simulink model "TosAeb"  (R2021a)
settings: Solver=FixedStepDiscrete  StartTime=0.0  StopTime=inf  FixedStep=0.01  RelTol=1e-3
undefined symbols (not defined in the file or in its folder): ACCModSts, AccSts1, AEB_ObjectMovingOrientation, AEB_ObjectMovingState, CN_BTCActive, CN_CurrentGearR, CN_CurrentGearR_DHT, CN_PTCActive, CN_VDCActive, CN_VSC_EspOff, CN_VSC_FCW_AEB_Error, CN_VSC_FCW_AEB_NoDispay, CN_VSC_FCW_AEB_PerfmDgdtn, CN_VSC_FCW_AEB_TempUnAvlbl, CN_VSC_VehStandstill, CNaccelAvoidMax, CNaccelAvoidMin, CNbrakePedalPress, CNcomfortStrLeftAccelMax, CNcomfortStrLeftAccelMin, CNcomfortStrRightAccelMax, CNcomfortStrRightAccelMin, CNDrvDoorOpen, CNDrvModeEco, CNDrvModeNorm, CNDrvModeOther, CNDrvModeSport, CNDrvSBRreminder, CNNull, CNobjInvalidID, CNPassDoorOpen, CNPassSBRreminder, CNPEBSAccelNegInf, CNPEBSAccelNull, CNPEBSTimeInf, CNPEBSTimeNull, CNSTOPMAX, CNSTOPNULL, CNsubjWidth, CNSwtOFF, CNSwtON, CNTTCMAX, CNTTCNULL, CNVehConfigAEBCycNone, CNVehConfigAEBNone, CNVehConfigAEBPedNone, CNVehConfigFCWNone, CNVehConfigJANone, CollisionSeverity, FALSE, FCWSnvty, JACycObjCalcInfoDefaultParams, JAPedObjCalcInfoDefaultParams, JAVehObjCalcInfoDefaultParams, latCycObjCalcInfoDefaultParams, latPedObjCalcInfoDefaultParams, longObjCalcInfoDefaultParams, ModSts, movingStatus, ObjectType ... (+1112)
libraries: simulink x3031, AEBSFunctionLib x50, Ta_Library x33, AEBSStateMachineLib x23, Mylibrary x12, simulink_extras x7, ObjSelect_lib x3, dspstat3 x2
(part 23 of 102)

== system TosAeb/FcwAebJa_Step_sys/ALG_AEBSystem/ALG_AEBController/AEB_FuncCondCalcApp/JaPedFunctionCondCalc/AEBJAPedAEBfullSuppAborCond/AEBJAPedAEBfullAborCondCalc ==
  inputSignals : Inport
  AEBJAPedAEBfullAborCondFinalJudge : Subsystem
  AEBJAPedAEBfullObjRelAborCondCalc : Subsystem
  AEBJAPedAEBfullVehRelAborCondCalc : Subsystem
  Bus Selector : BusSelector  OutputSignals=AEBJAPedTgtCalcInfo.JAPedTgtObjInfo.AEBJAPedObj.ObjectID
  Bus Selector1 : BusSelector  OutputSignals=vehCanInfo.SteerWheelSpd,vehCanInfo.AccrPedlPosnDiagc,vehStateCalcInfo.VSC_JAFuncSuppAborCond.VSC_JAPedVehAEBfullAborCond
  AEBJAPedAEBfullAborCondNoStandstillFulfilled : Outport
  AEBJAPedAEBfullAborCondStandstillFulfilled : Outport  Port=2
  wires:
  Bus Selector1/2 -> AEBJAPedAEBfullVehRelAborCondCalc/2(AccelPedalPositionDiag)  "<AccrPedlPosnDiagc>"
  Bus Selector1/1 -> AEBJAPedAEBfullVehRelAborCondCalc/1(SteerWheelSpd_DegS)  "<SteerWheelSpd>"
  AEBJAPedAEBfullAborCondFinalJudge/1(AEBJAPedAEBfullAborCondNoStndstillFulfilled) -> AEBJAPedAEBfullAborCondNoStandstillFulfilled/1
  AEBJAPedAEBfullVehRelAborCondCalc/1(AEBJAPedAEBfullVehRelAborCondFulfilled) -> AEBJAPedAEBfullAborCondFinalJudge/2(AEBJAPedAEBfullVehRelAborCondFulfilled)  "AEB_JaPedAEBfullVehRelAborCond"
  AEBJAPedAEBfullObjRelAborCondCalc/1(AEBJAPedAEBfullObjRelAborCondFulfilled) -> AEBJAPedAEBfullAborCondFinalJudge/1(AEBJAPedAEBfullObjRelAborCondFulfilled)  "AEB_JAPedAEBfullObjRelAborCond"
  inputSignals/1 -> Bus Selector1/1, Bus Selector/1
  Bus Selector/1 -> AEBJAPedAEBfullObjRelAborCondCalc/1(ObjectID)  "<ObjectID>"
  Bus Selector1/3 -> AEBJAPedAEBfullVehRelAborCondCalc/3(VSC_JAPedAEBfullAborCond)  "<VSC_JAPedVehAEBfullAborCond>"
  AEBJAPedAEBfullAborCondFinalJudge/2(AEBJAPedAEBfullAborCondStndstillFulfilled) -> AEBJAPedAEBfullAborCondStandstillFulfilled/1

== system TosAeb/FcwAebJa_Step_sys/ALG_AEBSystem/ALG_AEBController/AEB_FuncCondCalcApp/JaPedFunctionCondCalc/AEBJAPedAEBfullSuppAborCond/AEBJAPedAEBfullSuppCondCalc ==
  inputSignals : Inport
  AEBJAPedAEBfullSuppCondFinalJudge : Subsystem
  AEBJAPedAEBfullSuppObjCondCalcu : Subsystem
  AEBJAPedAEBfullSuppSituationCondCalc : Subsystem
  AEBJAPedAEBfullSuppVehCondCalc : Subsystem
  Bus Selector : BusSelector  OutputSignals=AEBJAPedTgtCalcInfo.JAPedTgtObjInfo.AEBJAPedObj.ObjectMovingOrientation,AEBJAPedTgtCalcInfo.JAPedTgtObjInfo.AEBJAPedObj.ObjectID,AEBJAPedTgtCalcInfo.JAPedTgtObjInfo.AEBJAPedObj.ObjectExistProb,AEBJAPedTgtCalcInfo.JAPedTgtObjInfo.AEBJAPedObj.ObjectIsObstacleProb,AEBJAPedTgtCalcInfo.JAPedTgtObjInfo.AEBJAPedObj.ObjectTypeCameraConfirmed,AEBJAPedTgtCalcInfo.JAPedTgtObjInfo.AEBJAPedObj.dy,AEBJAPedTgtCalcInfo.JAPedTgtObjInfo.AEBJAPedObjAbsInfo.vxAbsObj,AEBJAPedTgtCalcInfo.JAPedTgtObjInfo.AEBJAPedObjAbsInfo.vyRelCorr,AEBJAPedTgtCalcInfo.JAPedTgtObjInfo.AEBJAPedObjAbsInfo.ObjFusQuality
  Bus Selector1 : BusSelector  OutputSignals=subjInfo.subjVel,vehConfigInfo.VehConfigIbooster,vehCanInfo.ESP_MasterCylindBrakePress,vehCanInfo.AccrPedlPosnDiagc,vehCanInfo.SteerWheelAngle_Deg,vehCanInfo.LeftTurnSWSts,vehCanInfo.RightTurnSWSts,subjInfo.subjLatAccel,vehStateCalcInfo.VSC_JAFuncSuppAborCond.VSC_JAPedAEBfullSuppCond
  Bus Selector2 : BusSelector  OutputSignals=AEBJAPedTgtCalcInfo.JAPedCollisonAssessInfo.distBraking,AEBJAPedTgtCalcInfo.JAPedCollisonAssessInfo.distRadical2Collsion,AEBJAPedTgtCalcInfo.JAPedTgtObjInfo.AEBJAPedObjColliInfo.tPredictTime,AEBJAPedTgtCalcInfo.JAPedCollisonAssessInfo.TTB,AEBJAPedTgtCalcInfo.JAPedTgtObjInfo.AEBJAPedObjColliInfo.ObjColliFlg
  AEBJAPedAEBfullSuppCondFulfilled : Outport
  wires:
  Bus Selector1/2 -> AEBJAPedAEBfullSuppVehCondCalc/2(VehConfigIbooster)  "<VehConfigIbooster>"
  Bus Selector/9 -> AEBJAPedAEBfullSuppObjCondCalcu/9(ObjFusQuality)  "<ObjFusQuality>"
  Bus Selector/6 -> AEBJAPedAEBfullSuppObjCondCalcu/6(dy)  "<dy>"
  Bus Selector2/5 -> AEBJAPedAEBfullSuppSituationCondCalc/5(ObjColliFlg)  "<ObjColliFlg>"
  Bus Selector2/1 -> AEBJAPedAEBfullSuppSituationCondCalc/1(distBraking)  "<distBraking>"
  Bus Selector2/4 -> AEBJAPedAEBfullSuppSituationCondCalc/4(TTB)  "<TTB>"
  Bus Selector2/2 -> AEBJAPedAEBfullSuppSituationCondCalc/2(distRadical2Collsion)  "<distRadical2Collsion>"
  Bus Selector2/3 -> AEBJAPedAEBfullSuppSituationCondCalc/3(tPredictTime)  "<tPredictTime>"
  Bus Selector1/7 -> AEBJAPedAEBfullSuppVehCondCalc/7(RightTurnSWSts)  "<RightTurnSWSts>"
  Bus Selector1/8 -> AEBJAPedAEBfullSuppVehCondCalc/8(subjLatAccel)  "<subjLatAccel>"
  Bus Selector1/6 -> AEBJAPedAEBfullSuppVehCondCalc/6(LeftTurnSWSts)  "<LeftTurnSWSts>"
  Bus Selector1/5 -> AEBJAPedAEBfullSuppVehCondCalc/5(SteerWheelAngle_Deg)  "<SteerWheelAngle_Deg>"
  Bus Selector1/4 -> AEBJAPedAEBfullSuppVehCondCalc/4(AccrPedlPosnDiagc)  "<AccrPedlPosnDiagc>"
  Bus Selector1/3 -> AEBJAPedAEBfullSuppVehCondCalc/3(ESP_MasterCylindBrakePress)  "<ESP_MasterCylindBrakePress>"
  Bus Selector1/1 -> AEBJAPedAEBfullSuppVehCondCalc/1(subjVel)  "<subjVel>"
  Bus Selector/8 -> AEBJAPedAEBfullSuppObjCondCalcu/8(vyRelCorr)  "<vyRelCorr>"
  Bus Selector/7 -> AEBJAPedAEBfullSuppObjCondCalcu/7(vxAbsObj)  "<vxAbsObj>"
  Bus Selector/5 -> AEBJAPedAEBfullSuppObjCondCalcu/5(ObjectTypeCameraConfirmed)  "<ObjectTypeCameraConfirmed>"
  Bus Selector/4 -> AEBJAPedAEBfullSuppObjCondCalcu/4(ObjectIsObstacleProb)  "<ObjectIsObstacleProb>"
  Bus Selector/3 -> AEBJAPedAEBfullSuppObjCondCalcu/3(ObjectExistProb)  "<ObjectExistProb>"
  Bus Selector/2 -> AEBJAPedAEBfullSuppObjCondCalcu/2(ObjectID)  "<ObjectID>"
  Bus Selector/1 -> AEBJAPedAEBfullSuppObjCondCalcu/1(ObjectMovingOrientation)  "<ObjectMovingOrientation>"
  AEBJAPedAEBfullSuppSituationCondCalc/1(AEBJAPedAEBfullSuppSituationActiveCondFulfilled) -> AEBJAPedAEBfullSuppCondFinalJudge/3(AEBJAPedAEBfullSituationRelActiveCondFulfilled)  "AEB_JAPedAEBfullSitActiveCond"
  AEBJAPedAEBfullSuppObjCondCalcu/1(AEBJAPedAEBfullObjActiveCondFulfilled) -> AEBJAPedAEBfullSuppCondFinalJudge/1(AEBJAPedAEBfullObjRelActiveCondFulfilled)  "AEB_JaPedAEBfullObjActiveCond"
  AEBJAPedAEBfullSuppVehCondCalc/1(AEBJAPedAEBfullVehActiveCondFulfilled) -> AEBJAPedAEBfullSuppCondFinalJudge/2(AEBJAPedAEBfullVehRelActiveCondFulfilled)  "AEB_JaPedAEBfullVehActiveCond"
  AEBJAPedAEBfullSuppCondFinalJudge/1(AEBJAPedAEBfullSuppCondFulfilled) -> AEBJAPedAEBfullSuppCondFulfilled/1
  inputSignals/1 -> Bus Selector/1, Bus Selector1/1, Bus Selector2/1
  Bus Selector1/9 -> AEBJAPedAEBfullSuppVehCondCalc/9(VSC_JAPedAEBfullSuppCond)  "<VSC_JAPedAEBfullSuppCond>"

== system TosAeb/FcwAebJa_Step_sys/ALG_AEBSystem/ALG_AEBController/AEB_FuncCondCalcApp/JaPedFunctionCondCalc/AEBJAPedPrefillSuppAborCond/AEBJAPedPrefillAborCondCalc ==
  inputSignals : Inport
  AEBJAPedPrefillAborCondFinalJudge : Subsystem
  AEBJAPedPrefillObjRelAborCondCalc : Subsystem
  AEBJAPedPrefillSituationRelAborCondCalc : Subsystem
  AEBJAPedPrefillVehRelAborCondCalc : Subsystem
  Bus Selector : BusSelector  OutputSignals=AEBJAPedTgtCalcInfo.JAPedTgtObjInfo.AEBJAPedObj.ObjectID
  Bus Selector1 : BusSelector  OutputSignals=vehCanInfo.SteerWheelSpd_DegS,vehCanInfo.AccrPedlPosnDiagc,vehStateCalcInfo.VSC_JAFuncSuppAborCond.VSC_JAPedPrefillAborCond
  AEBJAPedPrefillAborCondFulfilled : Outport
  wires:
  Bus Selector1/3 -> AEBJAPedPrefillVehRelAborCondCalc/3(VSC_JAPedPrefillAborCond)  "<VSC_JAPedPrefillAborCond>"
  Bus Selector/1 -> AEBJAPedPrefillObjRelAborCondCalc/1(ObjectID)  "<ObjectID>"
  inputSignals/1 -> Bus Selector/1, Bus Selector1/1
  AEBJAPedPrefillObjRelAborCondCalc/1(AEBJAPedPrefillObjRelAborCondFulfilled) -> AEBJAPedPrefillAborCondFinalJudge/1(AEBJAPedPrefillObjRelAborCondFulfilled)  "AEB_JaPedPrefillObjRelAborCond"
  AEBJAPedPrefillVehRelAborCondCalc/1(AEBJAPedPrefillVehRelAborCondFulfilled) -> AEBJAPedPrefillAborCondFinalJudge/2(AEBJAPedPrefillVehRelAborCondFulfilled)  "AEB_JaPedPrefillVehRelAborCond"
  AEBJAPedPrefillSituationRelAborCondCalc/1(AEBJAPedPrefillSituationRelAborCondFulfilled) -> AEBJAPedPrefillAborCondFinalJudge/3(AEBJAPedPrefillSituationRelAborCondFulfilled)  "AEB_JaPedPrefillSitRelAborCond"
  AEBJAPedPrefillAborCondFinalJudge/1(AEBJAPedPrefillAborCondFulfilled) -> AEBJAPedPrefillAborCondFulfilled/1
  Bus Selector1/1 -> AEBJAPedPrefillVehRelAborCondCalc/1(SteerWheelSpd_DegS)  "<SteerWheelSpd_DegS>"
  Bus Selector1/2 -> AEBJAPedPrefillVehRelAborCondCalc/2(AccelPedalPositionDiag)  "<AccrPedlPosnDiagc>"

== system TosAeb/FcwAebJa_Step_sys/ALG_AEBSystem/ALG_AEBController/AEB_FuncCondCalcApp/JaPedFunctionCondCalc/AEBJAPedPrefillSuppAborCond/AEBJAPedPrefillSuppCondCalc ==
  inputSignals : Inport
  AEBJAPedPrefillSuppCondFinalJudge : Subsystem
  Bus Selector : BusSelector  OutputSignals=AEBJAPedTgtCalcInfo.JAPedTgtObjInfo.AEBJAPedObj.ObjectMovingOrientation,AEBJAPedTgtCalcInfo.JAPedTgtObjInfo.AEBJAPedObj.ObjectID,AEBJAPedTgtCalcInfo.JAPedTgtObjInfo.AEBJAPedObj.ObjectExistProb,AEBJAPedTgtCalcInfo.JAPedTgtObjInfo.AEBJAPedObj.ObjectIsObstacleProb,AEBJAPedTgtCalcInfo.JAPedTgtObjInfo.AEBJAPedObj.ObjectTypeCameraConfirmed,AEBJAPedTgtCalcInfo.JAPedTgtObjInfo.AEBJAPedObj.dy,AEBJAPedTgtCalcInfo.JAPedTgtObjInfo.AEBJAPedObjAbsInfo.vxAbsObj,AEBJAPedTgtCalcInfo.JAPedTgtObjInfo.AEBJAPedObjAbsInfo.vyRelCorr,AEBJAPedTgtCalcInfo.JAPedTgtObjInfo.AEBJAPedObjAbsInfo.ObjFusQuality
  Bus Selector1 : BusSelector  OutputSignals=subjInfo.subjVel,vehConfigInfo.VehConfigIbooster,vehCanInfo.BrakePedalStatus_G,vehCanInfo.ESP_MasterCylindBrakePress,vehCanInfo.IB_BrkPedalAppld,vehCanInfo.AccrPedlPosnDiagc,vehCanInfo.SteerWheelAngle_Deg,vehCanInfo.LeftTurnSWSts,vehCanInfo.RightTurnSWSts,subjInfo.subjLatAccel,vehStateCalcInfo.VSC_JAFuncSuppAborCond.VSC_JAPedPrefillSuppCond
  Bus Selector2 : BusSelector  OutputSignals=AEBJAPedTgtCalcInfo.JAPedCollisonAssessInfo.distBraking,AEBJAPedTgtCalcInfo.JAPedCollisonAssessInfo.distRadical2Collsion,AEBJAPedTgtCalcInfo.JAPedTgtObjInfo.AEBJAPedObjColliInfo.tPredictTime,AEBJAPedTgtCalcInfo.JAPedCollisonAssessInfo.TTB,AEBJAPedTgtCalcInfo.JAPedTgtObjInfo.AEBJAPedObjColliInfo.ObjColliFlg
  JaPedPrefillSuppSituationCondCalc : Subsystem
  JaPedPrefillSuppSubjCondCalcu : Subsystem
  JaPedhPrefillSuppObjCondCalcu : Subsystem
  AEBJAPedPrefillSuppCondFulfilled : Outport
  wires:
  Bus Selector1/2 -> JaPedPrefillSuppSubjCondCalcu/2(VehConfigIbooster)  "<VehConfigIbooster>"
  Bus Selector/9 -> JaPedhPrefillSuppObjCondCalcu/9(ObjFusQuality)  "<ObjFusQuality>"
  Bus Selector/6 -> JaPedhPrefillSuppObjCondCalcu/6(dy)  "<dy>"
  Bus Selector1/5 -> JaPedPrefillSuppSubjCondCalcu/5(IB_BrkPedalAppld)  "<IB_BrkPedalAppld>"
  Bus Selector2/1 -> JaPedPrefillSuppSituationCondCalc/1(distBraking)  "<distBraking>"
  Bus Selector2/2 -> JaPedPrefillSuppSituationCondCalc/2(distRadical2Collsion)  "<distRadical2Collsion>"
  Bus Selector1/9 -> JaPedPrefillSuppSubjCondCalcu/9(RightTurnSWSts)  "<RightTurnSWSts>"
  inputSignals/1 -> Bus Selector/1, Bus Selector1/1, Bus Selector2/1
  AEBJAPedPrefillSuppCondFinalJudge/1(AEBJAPedPrefillSuppCondFulfilled) -> AEBJAPedPrefillSuppCondFulfilled/1
  JaPedPrefillSuppSubjCondCalcu/1(AEBJAPedPrefillVehActiveCondFulfilled) -> AEBJAPedPrefillSuppCondFinalJudge/2(AEBJAPedPrefillVehRelActiveCondFulfilled)  "AEB_JaPedPrefillVehActiveCond"
  JaPedhPrefillSuppObjCondCalcu/1(AEBJAPedPrefillSuppObjActiveCondFulfilled) -> AEBJAPedPrefillSuppCondFinalJudge/1(AEBJAPedPrefillObjRelActiveCondFulfilled)  "AEB_JaPedPrefillObjActiveCond"
  JaPedPrefillSuppSituationCondCalc/1(AEBJAVehPrefillSuppSitActiveCondFulfilled) -> AEBJAPedPrefillSuppCondFinalJudge/3(AEBJAPedPrefillSituationRelActiveCondFulfilled)  "AEB_JaPrefillSitActiveCond"
  Bus Selector/1 -> JaPedhPrefillSuppObjCondCalcu/1(ObjectMovingOrientation)  "<ObjectMovingOrientation>"
  Bus Selector/2 -> JaPedhPrefillSuppObjCondCalcu/2(ObjectID)  "<ObjectID>"
  Bus Selector/3 -> JaPedhPrefillSuppObjCondCalcu/3(ObjectExistProb)  "<ObjectExistProb>"
  Bus Selector/4 -> JaPedhPrefillSuppObjCondCalcu/4(ObjectIsObstacleProb)  "<ObjectIsObstacleProb>"
  Bus Selector/5 -> JaPedhPrefillSuppObjCondCalcu/5(ObjectTypeCameraConfirmed)  "<ObjectTypeCameraConfirmed>"
  Bus Selector/7 -> JaPedhPrefillSuppObjCondCalcu/7(vxAbsObj)  "<vxAbsObj>"
  Bus Selector/8 -> JaPedhPrefillSuppObjCondCalcu/8(vyRelCorr)  "<vyRelCorr>"
  Bus Selector1/1 -> JaPedPrefillSuppSubjCondCalcu/1(subjVel)  "<subjVel>"
  Bus Selector1/3 -> JaPedPrefillSuppSubjCondCalcu/3(BrakePedalStatus_G)  "<BrakePedalStatus_G>"
  Bus Selector1/4 -> JaPedPrefillSuppSubjCondCalcu/4(ESP_MasterCylindBrakePress)  "<ESP_MasterCylindBrakePress>"
  Bus Selector1/6 -> JaPedPrefillSuppSubjCondCalcu/6(AccrPedlPosnDiagc)  "<AccrPedlPosnDiagc>"
  Bus Selector1/7 -> JaPedPrefillSuppSubjCondCalcu/7(SteerWheelAngle_Deg)  "<SteerWheelAngle_Deg>"
  Bus Selector1/8 -> JaPedPrefillSuppSubjCondCalcu/8(LeftTurnSWSts)  "<LeftTurnSWSts>"
  Bus Selector1/10 -> JaPedPrefillSuppSubjCondCalcu/10(subjLatAccel)  "<subjLatAccel>"
  Bus Selector2/3 -> JaPedPrefillSuppSituationCondCalc/3(tPredictTime)  "<tPredictTime>"
  Bus Selector2/4 -> JaPedPrefillSuppSituationCondCalc/4(TTB)  "<TTB>"
  Bus Selector2/5 -> JaPedPrefillSuppSituationCondCalc/5(ObjColliFlg)  "<ObjColliFlg>"
  Bus Selector1/11 -> JaPedPrefillSuppSubjCondCalcu/11(VSC_JAPedPrefillSuppCond)  "<VSC_JAPedPrefillSuppCond>"

== system TosAeb/FcwAebJa_Step_sys/ALG_AEBSystem/ALG_AEBController/AEB_FuncCondCalcApp/JaVehFunctionCondCalc/AEBJAVehAEBfullSuppAborCond/AEBJAVehAEBfullAborCondCalc ==
  inputSignals : Inport
  AEBJAVehAEBfullAborCondFinalJudge : Subsystem
  AEBJAVehAEBfullObjRelAborCondCalc : Subsystem
  AEBJAVehAEBfullVehRelAborCondCalc : Subsystem
  Bus Selector : BusSelector  OutputSignals=AEBJAVehTgtCalcInfo.JAVehTgtObjInfo.AEBJAVehObj.ObjectID
  Bus Selector1 : BusSelector  OutputSignals=vehCanInfo.SteerWheelAngleSign,vehCanInfo.SteerWheelSpd_DegS,vehCanInfo.AccrPedlPosnDiagc,vehStateCalcInfo.VSC_JAFuncSuppAborCond.VSC_JAVehAEBfullAborCond
  AEBJAVehAEBfullAborCondNoStandstillFulfilled : Outport
  AEBJAVehAEBfullAborCondStandstillFulfilled : Outport  Port=2
  wires:
  AEBJAVehAEBfullAborCondFinalJudge/2(AEBJAVehAEBfullAborCondStndstillFulfilled) -> AEBJAVehAEBfullAborCondStandstillFulfilled/1
  Bus Selector1/4 -> AEBJAVehAEBfullVehRelAborCondCalc/4(VSC_JAVehAEBfullAborCond)  "<VSC_JAVehAEBfullAborCond>"
  Bus Selector/1 -> AEBJAVehAEBfullObjRelAborCondCalc/1(ObjectID)  "<ObjectID>"
  inputSignals/1 -> Bus Selector/1, Bus Selector1/1
  AEBJAVehAEBfullObjRelAborCondCalc/1(AEBJAVehAEBfullObjRelAborCondFulfilled) -> AEBJAVehAEBfullAborCondFinalJudge/1(AEBJAVehAEBfullObjRelAborCondFulfilled)  "AEB_JaVehAEBfullObjRelAborCond"
  AEBJAVehAEBfullVehRelAborCondCalc/1(AEBJAVehAEBfullVehRelAborCondFulfilled) -> AEBJAVehAEBfullAborCondFinalJudge/2(AEBJAVehAEBfullVehRelAborCondFulfilled)  "AEB_JaVehAEBfullVehRelAborCond"
  AEBJAVehAEBfullAborCondFinalJudge/1(AEBJAVehAEBfullAborCondNoStndstillFulfilled) -> AEBJAVehAEBfullAborCondNoStandstillFulfilled/1
  Bus Selector1/1 -> AEBJAVehAEBfullVehRelAborCondCalc/1(SteerWheelAngleSign)  "<SteerWheelAngleSign>"
  Bus Selector1/2 -> AEBJAVehAEBfullVehRelAborCondCalc/2(SteerWheelSpd_DegS)  "<SteerWheelSpd_DegS>"
  Bus Selector1/3 -> AEBJAVehAEBfullVehRelAborCondCalc/3(AccelPedalPositionDiag)  "<AccrPedlPosnDiagc>"

== system TosAeb/FcwAebJa_Step_sys/ALG_AEBSystem/ALG_AEBController/AEB_FuncCondCalcApp/JaVehFunctionCondCalc/AEBJAVehAEBfullSuppAborCond/AEBJAVehAEBfullSuppCondCalc ==
  inputSignals : Inport
  AEBJAVehAEBfullSuppCondFinalJudge : Subsystem
  AEBJAVehAEBfullSuppObjCondCalcu : Subsystem
  AEBJAVehAEBfullSuppSituationCondCalc : Subsystem
  AEBJAVehAEBfullSuppSubjCondCalcu : Subsystem
  Bus Selector : BusSelector  OutputSignals=AEBJAVehTgtCalcInfo.JAVehTgtObjInfo.AEBJAVehObj.ObjectMovingState,AEBJAVehTgtCalcInfo.JAVehTgtObjInfo.AEBJAVehObj.ObjectMovingOrientation,AEBJAVehTgtCalcInfo.JAVehTgtObjInfo.AEBJAVehObj.ObjectID,AEBJAVehTgtCalcInfo.JAVehTgtObjInfo.AEBJAVehObj.ObjectExistProb,AEBJAVehTgtCalcInfo.JAVehTgtObjInfo.AEBJAVehObj.ObjectIsObstacleProb,AEBJAVehTgtCalcInfo.JAVehTgtObjInfo.AEBJAVehObj.ObjectTypeCameraConfirmed,AEBJAVehTgtCalcInfo.JAVehTgtObjInfo.AEBJAVehObj.dy,AEBJAVehTgtCalcInfo.JAVehTgtObjInfo.AEBJAVehObjAbsInfo.vxAbsObj,AEBJAVehTgtCalcInfo.JAVehTgtObjInfo.AEBJAVehObjAbsInfo.vyRelCorr,AEBJAVehTgtCalcInfo.JAVehTgtObjInfo.AEBJAVehObjAbsInfo.ObjFusQuality
  Bus Selector1 : BusSelector  OutputSignals=subjInfo.subjVel,vehConfigInfo.VehConfigIbooster,vehCanInfo.ESP_MasterCylindBrakePress,vehCanInfo.AccrPedlPosnDiagc,vehCanInfo.SteerWheelAngle_Deg,vehCanInfo.LeftTurnSWSts,subjInfo.subjLatAccel,vehStateCalcInfo.VSC_JAFuncSuppAborCond.VSC_JAVehAEBfullSuppCond
  Bus Selector2 : BusSelector  OutputSignals=AEBJAVehTgtCalcInfo.JAVehCollisonAssessInfo.initialLatDist,AEBJAVehTgtCalcInfo.JAVehCollisonAssessInfo.restLatDist2Collsion,AEBJAVehTgtCalcInfo.JAVehTgtObjInfo.AEBJAVehObjColliInfo.tPredictTime,AEBJAVehTgtCalcInfo.JAVehCollisonAssessInfo.TTB,AEBJAVehTgtCalcInfo.JAVehTgtObjInfo.AEBJAVehObjColliInfo.ObjColliFlg
  AEBJAVehAEBfullSuppCondFulfilled : Outport
  wires:
  Bus Selector1/2 -> AEBJAVehAEBfullSuppSubjCondCalcu/2(VehConfigIbooster)  "<VehConfigIbooster>"
  Bus Selector/10 -> AEBJAVehAEBfullSuppObjCondCalcu/10(ObjFusQuality)  "<ObjFusQuality>"
  Bus Selector/3 -> AEBJAVehAEBfullSuppObjCondCalcu/3(ObjectID)  "<ObjectID>"
  Bus Selector/1 -> AEBJAVehAEBfullSuppObjCondCalcu/1(ObjectMovingState)  "<ObjectMovingState>"
  Bus Selector/5 -> AEBJAVehAEBfullSuppObjCondCalcu/5(ObjectIsObstacleProb)  "<ObjectIsObstacleProb>"
  Bus Selector/2 -> AEBJAVehAEBfullSuppObjCondCalcu/2(ObjectMovingOrientation)  "<ObjectMovingOrientation>"
  Bus Selector/8 -> AEBJAVehAEBfullSuppObjCondCalcu/8(vxAbsObj)  "<vxAbsObj>"
  Bus Selector/7 -> AEBJAVehAEBfullSuppObjCondCalcu/7(dy)  "<dy>"
  Bus Selector/6 -> AEBJAVehAEBfullSuppObjCondCalcu/6(ObjectTypeCameraConfirmed)  "<ObjectTypeCameraConfirmed>"
  Bus Selector/9 -> AEBJAVehAEBfullSuppObjCondCalcu/9(vyRelCorr)  "<vyRelCorr>"
  Bus Selector/4 -> AEBJAVehAEBfullSuppObjCondCalcu/4(ObjectExistProb)  "<ObjectExistProb>"
  Bus Selector1/1 -> AEBJAVehAEBfullSuppSubjCondCalcu/1(subjVel)  "<subjVel>"
  Bus Selector1/3 -> AEBJAVehAEBfullSuppSubjCondCalcu/3(ESP_MasterCylindBrakePress)  "<ESP_MasterCylindBrakePress>"
  Bus Selector1/8 -> AEBJAVehAEBfullSuppSubjCondCalcu/8(VSC_JAVehAEBfullSuppCond)  "<VSC_JAVehAEBfullSuppCond>"
  Bus Selector1/6 -> AEBJAVehAEBfullSuppSubjCondCalcu/6(LeftTurnSWSts)  "<LeftTurnSWSts>"
  Bus Selector1/4 -> AEBJAVehAEBfullSuppSubjCondCalcu/4(AccrPedlPosnDiagc)  "<AccrPedlPosnDiagc>"
  Bus Selector1/5 -> AEBJAVehAEBfullSuppSubjCondCalcu/5(SteerWheelAngle_Deg)  "<SteerWheelAngle_Deg>"
  Bus Selector1/7 -> AEBJAVehAEBfullSuppSubjCondCalcu/7(LateralAcc)  "<subjLatAccel>"
  Bus Selector2/4 -> AEBJAVehAEBfullSuppSituationCondCalc/4(TTB)  "<TTB>"
  Bus Selector2/3 -> AEBJAVehAEBfullSuppSituationCondCalc/3(tPredictTime)  "<tPredictTime>"
  Bus Selector2/5 -> AEBJAVehAEBfullSuppSituationCondCalc/5(ObjColliFlg)  "<ObjColliFlg>"
  Bus Selector2/1 -> AEBJAVehAEBfullSuppSituationCondCalc/1(initialLatDist)  "<initialLatDist>"
  Bus Selector2/2 -> AEBJAVehAEBfullSuppSituationCondCalc/2(restLatDist2Collsion)  "<restLatDist2Collsion>"
  inputSignals/1 -> Bus Selector1/1, Bus Selector2/1, Bus Selector/1
  AEBJAVehAEBfullSuppCondFinalJudge/1(AEBJAVehAEBfullSuppCondFulfilled) -> AEBJAVehAEBfullSuppCondFulfilled/1
  AEBJAVehAEBfullSuppSubjCondCalcu/1(AEBJAVehAEBfullVehActiveCondFulfilled) -> AEBJAVehAEBfullSuppCondFinalJudge/2(AEBJAVehAEBfullVehRelActiveCondFulfilled)  "AEB_JaVehAEBfullVehActiveCond"
  AEBJAVehAEBfullSuppObjCondCalcu/1(AEBJAVehAEBfullObjActiveCondFulfilled) -> AEBJAVehAEBfullSuppCondFinalJudge/1(AEBJAVehAEBfullObjRelActiveCondFulfilled)  "AEB_JaVehAEBfullObjActiveCond"
  AEBJAVehAEBfullSuppSituationCondCalc/1(AEBJAVehAEBfullActiveSituationCondFulfilled) -> AEBJAVehAEBfullSuppCondFinalJudge/3(AEBJAVehAEBfullSituationRelActiveCondFulfilled)  "AEB_JaVehAEBfullSitActiveCond"

== system TosAeb/FcwAebJa_Step_sys/ALG_AEBSystem/ALG_AEBController/AEB_FuncCondCalcApp/JaVehFunctionCondCalc/AEBJAVehPrefillSuppAborCond/AEBJAVehPrefillAborCondCalc ==
  inputSignals : Inport
  AEBJAVehPrefillObjRelAborCondCalc : Subsystem
  AEBJAVehPrefillSituationRelAborCondCalc : Subsystem
  AEBJAVehPrefillVehRelAborCondCalc : Subsystem
  Bus Selector : BusSelector  OutputSignals=AEBJAVehTgtCalcInfo.JAVehTgtObjInfo.AEBJAVehObj.ObjectID
  Bus Selector1 : BusSelector  OutputSignals=vehCanInfo.SteerWheelAngleSign,vehCanInfo.SteerWheelSpd_DegS,vehCanInfo.AccrPedlPosnDiagc
  Bus Selector2 : BusSelector  OutputSignals=vehStateCalcInfo.VSC_JAFuncSuppAborCond.VSC_JAVehPrefillAborCond
  pedPrefillAborCondFinalJudge : Subsystem
  AEBJAVehPrefillAborCondFulfilled : Outport
  wires:
  Bus Selector1/3 -> AEBJAVehPrefillVehRelAborCondCalc/3(AccelPedalPositionDiag)  "<AccrPedlPosnDiagc>"
  Bus Selector1/2 -> AEBJAVehPrefillVehRelAborCondCalc/2(SteerWheelSpd_degS)  "<SteerWheelSpd_DegS>"
  Bus Selector1/1 -> AEBJAVehPrefillVehRelAborCondCalc/1(SteerWheelAngleSign)  "<SteerWheelAngleSign>"
  pedPrefillAborCondFinalJudge/1(AEBJAVehPrefillAborCondFulfilled) -> AEBJAVehPrefillAborCondFulfilled/1
  AEBJAVehPrefillSituationRelAborCondCalc/1(AEBJAVehPrefillSituationRelAborCondFulfilled) -> pedPrefillAborCondFinalJudge/3(AEBJAVehPrefillSituationRelAborCondFulfilled)  "AEB_JaVehPrefillSitRelAborCond"
  AEBJAVehPrefillVehRelAborCondCalc/1(AEBJAVehPrefillVehRelAborCondFulfilled) -> pedPrefillAborCondFinalJudge/2(AEBJAVehPrefillVehRelAborCondFulfilled)  "AEB_JaVehPrefillVehRelAborCond"
  AEBJAVehPrefillObjRelAborCondCalc/1(AEBJAVehPrefillObjRelAborCondFulfilled) -> pedPrefillAborCondFinalJudge/1(AEBJAVehPrefillObjRelAborCondFulfilled)  "AEB_JaVehPrefillObjRelAborCond"
  inputSignals/1 -> Bus Selector1/1, Bus Selector2/1, Bus Selector/1
  Bus Selector/1 -> AEBJAVehPrefillObjRelAborCondCalc/1(ObjectID)  "<ObjectID>"
  Bus Selector2/1 -> AEBJAVehPrefillSituationRelAborCondCalc/1(VSC_JAVehPrefillAborCond)  "<VSC_JAVehPrefillAborCond>"

== system TosAeb/FcwAebJa_Step_sys/ALG_AEBSystem/ALG_AEBController/AEB_FuncCondCalcApp/JaVehFunctionCondCalc/AEBJAVehPrefillSuppAborCond/AEBJAVehPrefillSuppCondCalc ==
  inputSignals : Inport
  AEBJAVehPrefillSuppCondFinalJudge : Subsystem
  AEBJAVehPrefillSuppObjCondCalcu : Subsystem
  AEBJAVehPrefillSuppSituationCondCalc : Subsystem
  AEBJAVehPrefillSuppSubjCondCalcu : Subsystem
  Bus Selector : BusSelector  OutputSignals=AEBJAVehTgtCalcInfo.JAVehTgtObjInfo.AEBJAVehObj.ObjectMovingState,AEBJAVehTgtCalcInfo.JAVehTgtObjInfo.AEBJAVehObj.ObjectMovingOrientation,AEBJAVehTgtCalcInfo.JAVehTgtObjInfo.AEBJAVehObj.ObjectID,AEBJAVehTgtCalcInfo.JAVehTgtObjInfo.AEBJAVehObj.ObjectExistProb,AEBJAVehTgtCalcInfo.JAVehTgtObjInfo.AEBJAVehObj.ObjectIsObstacleProb,AEBJAVehTgtCalcInfo.JAVehTgtObjInfo.AEBJAVehObj.ObjectTypeCameraConfirmed,AEBJAVehTgtCalcInfo.JAVehTgtObjInfo.AEBJAVehObj.dy,AEBJAVehTgtCalcInfo.JAVehTgtObjInfo.AEBJAVehObjAbsInfo.vxAbsObj,AEBJAVehTgtCalcInfo.JAVehTgtObjInfo.AEBJAVehObjAbsInfo.vyRelCorr,AEBJAVehTgtCalcInfo.JAVehTgtObjInfo.AEBJAVehObjAbsInfo.ObjFusQuality
  Bus Selector1 : BusSelector  OutputSignals=subjInfo.subjVel,vehConfigInfo.VehConfigIbooster,vehCanInfo.ESP_MasterCylindBrakePress,vehCanInfo.BrakePedalStatus_G,vehCanInfo.IB_BrkPedalAppld,vehCanInfo.AccrPedlPosnDiagc,vehCanInfo.SteerWheelAngle_Deg,vehCanInfo.LeftTurnSWSts,subjInfo.subjLatAccel,vehStateCalcInfo.VSC_JAFuncSuppAborCond.VSC_JAVehPrefillSuppCond
  Bus Selector2 : BusSelector  OutputSignals=AEBJAVehTgtCalcInfo.JAVehCollisonAssessInfo.initialLatDist,AEBJAVehTgtCalcInfo.JAVehCollisonAssessInfo.restLatDist2Collsion,AEBJAVehTgtCalcInfo.JAVehTgtObjInfo.AEBJAVehObjColliInfo.tPredictTime,AEBJAVehTgtCalcInfo.JAVehCollisonAssessInfo.TTB,AEBJAVehTgtCalcInfo.JAVehTgtObjInfo.AEBJAVehObjColliInfo.ObjColliFlg
  AEBJAVehPrefillSuppCondFulfilled : Outport
  wires:
  Bus Selector1/2 -> AEBJAVehPrefillSuppSubjCondCalcu/2(VehConfigIbooster)  "<VehConfigIbooster>"
  Bus Selector/10 -> AEBJAVehPrefillSuppObjCondCalcu/10(ObjFusQuality)  "<ObjFusQuality>"
  Bus Selector/6 -> AEBJAVehPrefillSuppObjCondCalcu/6(ObjectTypeCameraConfirmed)  "<ObjectTypeCameraConfirmed>"
  Bus Selector/1 -> AEBJAVehPrefillSuppObjCondCalcu/1(ObjectMovingState)  "<ObjectMovingState>"
  Bus Selector/5 -> AEBJAVehPrefillSuppObjCondCalcu/5(ObjectIsObstacleProb)  "<ObjectIsObstacleProb>"
  Bus Selector/9 -> AEBJAVehPrefillSuppObjCondCalcu/9(vyRelCorr)  "<vyRelCorr>"
  Bus Selector/3 -> AEBJAVehPrefillSuppObjCondCalcu/3(ObjectID)  "<ObjectID>"
  Bus Selector/2 -> AEBJAVehPrefillSuppObjCondCalcu/2(ObjectMovingOrientation)  "<ObjectMovingOrientation>"
  Bus Selector/4 -> AEBJAVehPrefillSuppObjCondCalcu/4(ObjectExistProb)  "<ObjectExistProb>"
  Bus Selector/7 -> AEBJAVehPrefillSuppObjCondCalcu/7(dy)  "<dy>"
  Bus Selector/8 -> AEBJAVehPrefillSuppObjCondCalcu/8(vxAbsObj)  "<vxAbsObj>"
  Bus Selector1/1 -> AEBJAVehPrefillSuppSubjCondCalcu/1(subjVel)  "<subjVel>"
  Bus Selector1/4 -> AEBJAVehPrefillSuppSubjCondCalcu/4(BrakePedalStatus_G)  "<BrakePedalStatus_G>"
  Bus Selector1/8 -> AEBJAVehPrefillSuppSubjCondCalcu/8(LeftTurnSWSts)  "<LeftTurnSWSts>"
  Bus Selector1/7 -> AEBJAVehPrefillSuppSubjCondCalcu/7(SteerWheelAngle_Deg)  "<SteerWheelAngle_Deg>"
  Bus Selector1/3 -> AEBJAVehPrefillSuppSubjCondCalcu/3(ESP_MasterCylindBrakePress)  "<ESP_MasterCylindBrakePress>"
  Bus Selector1/5 -> AEBJAVehPrefillSuppSubjCondCalcu/5(IB_BrkPedalAppld)  "<IB_BrkPedalAppld>"
  Bus Selector1/6 -> AEBJAVehPrefillSuppSubjCondCalcu/6(AccrPedlPosnDiagc)  "<AccrPedlPosnDiagc>"
  Bus Selector1/9 -> AEBJAVehPrefillSuppSubjCondCalcu/9(subjLatAccel)  "<subjLatAccel>"
  Bus Selector1/10 -> AEBJAVehPrefillSuppSubjCondCalcu/10(VSC_JAVehPrefillSuppCond)  "<VSC_JAVehPrefillSuppCond>"
  Bus Selector2/4 -> AEBJAVehPrefillSuppSituationCondCalc/4(TTB)  "<TTB>"
  Bus Selector2/3 -> AEBJAVehPrefillSuppSituationCondCalc/3(tPredictTime)  "<tPredictTime>"
  Bus Selector2/2 -> AEBJAVehPrefillSuppSituationCondCalc/2(restLatDist2Collision)  "<restLatDist2Collsion>"
  Bus Selector2/1 -> AEBJAVehPrefillSuppSituationCondCalc/1(initialLatDist)  "<initialLatDist>"
  Bus Selector2/5 -> AEBJAVehPrefillSuppSituationCondCalc/5(ObjColliFlg)  "<ObjColliFlg>"
  AEBJAVehPrefillSuppSituationCondCalc/1(AEBJAVehPrefillActiveSituationCondFulfilled) -> AEBJAVehPrefillSuppCondFinalJudge/3(AEBJAVehPrefillSituationRelActiveCondFulfilled)  "AEB_JaVehPrefillActiveSitCond"
  AEBJAVehPrefillSuppObjCondCalcu/1(AEBJAVehPrefillSuppObjActiveCondFulfilled) -> AEBJAVehPrefillSuppCondFinalJudge/1(AEBJAVehPrefillObjRelActiveCondFulfilled)  "AEB_JaVehPrefillObjActiveCond"
  AEBJAVehPrefillSuppSubjCondCalcu/1(AEBJAVehPrefillVehActiveCondFulfilled) -> AEBJAVehPrefillSuppCondFinalJudge/2(AEBJAVehPrefillVehRelActiveCondFulfilled)  "AEB_JaVehPrefillVehActiveCond"
  AEBJAVehPrefillSuppCondFinalJudge/1(AEBJAVehPrefillSuppCondFulfilled) -> AEBJAVehPrefillSuppCondFulfilled/1
  inputSignals/1 -> Bus Selector1/1, Bus Selector2/1, Bus Selector/1

== system TosAeb/FcwAebJa_Step_sys/ALG_AEBSystem/ALG_AEBController/AEB_FuncCondCalcApp/LongFunctionCondCalc/vehABASuppAborCondCalc/vehABAAborCondCalc ==
  inputSignals : Inport
  Bus Selector : BusSelector  OutputSignals=longObjCalcInfo.LongObjRawInfo.ObjectID
  Bus Selector1 : BusSelector  OutputSignals=vehCanInfo.SteerWheelAngle,vehCanInfo.SteerWheelSpd,vehCanInfo.AccrPedlPosnDiagc,vehStateCalcInfo.VSCVehSuppAborCond.VSCvehABAAborCond
  Bus Selector2 : BusSelector  OutputSignals=longObjCalcInfo.LongCollisionAssess.longCollisionSeverity
  vehABAAborCondFinalJudge : Subsystem
  vehABAObjRelAborCondCalc : Subsystem
  vehABASituationRelAborCondCalc : Subsystem
  vehABAVehRelAborCondCalc : Subsystem
  vehABAAborCondFulfilled : Outport
  wires:
  Bus Selector2/1 -> vehABASituationRelAborCondCalc/1(longCollisionSeverity)  "<longCollisionSeverity>"
  Bus Selector1/1 -> vehABAVehRelAborCondCalc/1(SteerWheelAngle)  "<SteerWheelAngle>"
  Bus Selector1/4 -> vehABAVehRelAborCondCalc/4(vehStateCalcABAAborFlg)  "<VSCvehABAAborCond>"
  Bus Selector1/2 -> vehABAVehRelAborCondCalc/2(SteerWheelSpd)  "<SteerWheelSpd>"
  Bus Selector1/3 -> vehABAVehRelAborCondCalc/3(AccrPedlPosnDiagc)  "<AccrPedlPosnDiagc>"
  inputSignals/1 -> Bus Selector/1, Bus Selector1/1, Bus Selector2/1
  Bus Selector/1 -> vehABAObjRelAborCondCalc/1(ObjectID)  "<ObjectID>"
  vehABAObjRelAborCondCalc/1(vehABAObjRelAborCondFulfilled) -> vehABAAborCondFinalJudge/1(vehABAObjRelAborCondFulfilled)  "AEB_vehABAObjRelAborCond"
  vehABAVehRelAborCondCalc/1(vehABAVehRelAborCondFulfilled) -> vehABAAborCondFinalJudge/2(vehABAVehRelAborCondFulfilled)  "AEB_vehABAVehRelAborCond"
  vehABASituationRelAborCondCalc/1(vehABASituationRelAborCondFulfilled) -> vehABAAborCondFinalJudge/3(vehABASituationRelAborCondFulfilled)  "AEB_vehABASitRelAborCond"
  vehABAAborCondFinalJudge/1(vehABAAborCondFulfilled) -> vehABAAborCondFulfilled/1

== system TosAeb/FcwAebJa_Step_sys/ALG_AEBSystem/ALG_AEBController/AEB_FuncCondCalcApp/LongFunctionCondCalc/vehABASuppAborCondCalc/vehABASuppCondCalc ==
  inputSignals : Inport
  Bus Selector : BusSelector  OutputSignals=longObjCalcInfo.LongObjRawInfo.ObjectMovingState,longObjCalcInfo.LongObjRawInfo.ObjectTypeCameraConfirmed,vehStateCalcInfo.VSC_AEBFuncSwtSts.AEB_PedResp,longObjCalcInfo.LongObjRawInfo.ObjectType,subjInfo.subjCurvTraj,subjInfo.subjLatAccel,subjInfo.subjVel,vehConfigInfo.VehicleConfigAEBCyc,vehConfigInfo.VehicleConfigAEBPed,longObjCalcInfo.LongObjRawInfo.ObjectMovingOrientation,longObjCalcInfo.LongObjRawInfo.ObjectID,longObjCalcInfo.LongObjRawInfo.ObjectExistProb,longObjCalcInfo.LongObjRawInfo.ObjectIsObstacleProb,longObjCalcInfo.longObjAbsInfo.ObjFusQuality
  Bus Selector1 : BusSelector  OutputSignals=subjInfo.subjVel,longObjCalcInfo.LongObjRawInfo.ObjectMovingState,vehStateCalcInfo.VSCVehSuppAborCond.VSCvehABASuppCond,vehCanInfo.SteerWheelAngle,vehCanInfo.SteerWheelSpd,vehCanInfo.AccrPedlPosnDiagc
  Bus Selector2 : BusSelector  OutputSignals=longObjCalcInfo.LongCollisionAssess.longCollisionSeverity,longObjCalcInfo.overlapRatio,longObjCalcInfo.longObjAbsInfo.dxFrontBumper,longObjCalcInfo.probEscape
  vehABAObjRelActiveCondCalc : Subsystem
  vehABASituationRelActiveCondCalc : Subsystem
  vehABASuppCondFinalJudge : Subsystem
  vehABAVehRelActiveCondCalc : Subsystem
  vehABASuppCondFulfilled : Outport
  wires:
  Bus Selector/14 -> vehABAObjRelActiveCondCalc/14(ObjFusQuality)  "<ObjFusQuality>"
  Bus Selector2/1 -> vehABASituationRelActiveCondCalc/1(longCollisionSeverity)  "<longCollisionSeverity>"
  Bus Selector2/4 -> vehABASituationRelActiveCondCalc/4(probEscape)  "<probEscape>"
  Bus Selector2/2 -> vehABASituationRelActiveCondCalc/2(overlapRatio)  "<overlapRatio>"
  Bus Selector2/3 -> vehABASituationRelActiveCondCalc/3(dxFrontBumper)  "<dxFrontBumper>"
  Bus Selector1/1 -> vehABAVehRelActiveCondCalc/1(subjVel)  "<subjVel>"
  Bus Selector1/6 -> vehABAVehRelActiveCondCalc/6(AccrPedlPosnDiagc)  "<AccrPedlPosnDiagc>"
  Bus Selector1/2 -> vehABAVehRelActiveCondCalc/2(ObjectMovingState)  "<ObjectMovingState>"
  Bus Selector1/5 -> vehABAVehRelActiveCondCalc/5(SteerWheelSpd)  "<SteerWheelSpd>"
  Bus Selector1/3 -> vehABAVehRelActiveCondCalc/3(vehStateCalcABASuppFlg)  "<VSCvehABASuppCond>"
  Bus Selector1/4 -> vehABAVehRelActiveCondCalc/4(SteerWheelAngle)  "<SteerWheelAngle>"
  Bus Selector/13 -> vehABAObjRelActiveCondCalc/13(ObjectIsObstacleProb)  "<ObjectIsObstacleProb>"
  Bus Selector/12 -> vehABAObjRelActiveCondCalc/12(ObjectExistProb)  "<ObjectExistProb>"
  Bus Selector/11 -> vehABAObjRelActiveCondCalc/11(ObjectID)  "<ObjectID>"
  Bus Selector/10 -> vehABAObjRelActiveCondCalc/10(ObjectMovingOrientation)  "<ObjectMovingOrientation>"
  Bus Selector/9 -> vehABAObjRelActiveCondCalc/9(vehConfigAEBPed)  "<VehicleConfigAEBPed>"
  Bus Selector/8 -> vehABAObjRelActiveCondCalc/8(vehConfigAEBCyc)  "<VehicleConfigAEBCyc>"
  Bus Selector/7 -> vehABAObjRelActiveCondCalc/7(subjVel)  "<subjVel>"
  Bus Selector/6 -> vehABAObjRelActiveCondCalc/6(subjLatAccel)  "<subjLatAccel>"
  Bus Selector/5 -> vehABAObjRelActiveCondCalc/5(subjCurvTraj)  "<subjCurvTraj>"
  Bus Selector/4 -> vehABAObjRelActiveCondCalc/4(ObjectType)  "<ObjectType>"
  Bus Selector/3 -> vehABAObjRelActiveCondCalc/3(AEB_PedResp)  "<AEB_PedResp>"
  Bus Selector/2 -> vehABAObjRelActiveCondCalc/2(ObjectTypeCameraConfirmed)  "<ObjectTypeCameraConfirmed>"
  Bus Selector/1 -> vehABAObjRelActiveCondCalc/1(ObjectMovingState)  "<ObjectMovingState>"
  inputSignals/1 -> Bus Selector/1, Bus Selector1/1, Bus Selector2/1
  vehABASuppCondFinalJudge/1(vehABASuppCondFulfilled) -> vehABASuppCondFulfilled/1  "vehAEBfullSuppCondFulfilled"
  vehABAVehRelActiveCondCalc/1(vehABAVehRelActiveCondFulfilled) -> vehABASuppCondFinalJudge/2(vehABAVehRelActiveCondFulfilled)  "AEB_vehABAVehRelActiveCond"
  vehABAObjRelActiveCondCalc/1(vehABAObjRelActiveCondFulfilled) -> vehABASuppCondFinalJudge/1(vehABAObjRelActiveCondFulfilled)  "AEB_vehABAObjRelActiveCond"
  vehABASituationRelActiveCondCalc/1(vehABASituationRelActiveCondFulfilled) -> vehABASuppCondFinalJudge/3(vehABASituationRelActiveCondFulfilled)  "AEB_vehABASitRelActiveCond"

== system TosAeb/FcwAebJa_Step_sys/ALG_AEBSystem/ALG_AEBController/AEB_FuncCondCalcApp/LongFunctionCondCalc/vehAEBfullSuppAborCondCalc/ vehAEBfullSuppCondCalc ==
  inputSignals : Inport
  Bus Selector : BusSelector  OutputSignals=longObjCalcInfo.LongObjRawInfo.ObjectMovingState,longObjCalcInfo.LongObjRawInfo.dz,longObjCalcInfo.longObjAbsInfo.dxFrontBumper,longObjCalcInfo.LongObjRawInfo.ObjectTypeCameraConfirmed,longObjCalcInfo.LongObjRawInfo.ObjectType,subjInfo.subjCurvTraj,subjInfo.subjLatAccel,subjInfo.subjVel,vehConfigInfo.VehicleConfigAEBCyc,vehConfigInfo.VehicleConfigAEBPed,vehStateCalcInfo.VSC_AEBFuncSwtSts.AEB_PedResp,longObjCalcInfo.LongObjRawInfo.ObjectMovingOrientation,longObjCalcInfo.LongObjRawInfo.ObjectID,longObjCalcInfo.LongObjRawInfo.ObjectExistProb,longObjCalcInfo.LongObjRawInfo.ObjectIsObstacleProb,longObjCalcInfo.LongObjRawInfo.dxVariance,longObjCalcInfo.longObjAbsInfo.ObjFusQuality
  Bus Selector1 : BusSelector  OutputSignals=longObjCalcInfo.LongObjRawInfo.ObjectTypeCameraConfirmed,longObjCalcInfo.LongObjRawInfo.ObjectMovingState,vehStateCalcInfo.VSCVehSuppAborCond.VSCvehAEBfullSuppCond,vehCanInfo.SteerWheelAngle,vehCanInfo.SteerWheelSpd,vehConfigInfo.VehConfigIbooster,vehCanInfo.ESP_MasterCylindBrakePress,vehCanInfo.BrakePedalStatus_G,subjInfo.subjLongAccel,subjInfo.subjVel,vehCanInfo.AccrPedlPosnDiagc
  Bus Selector2 : BusSelector  OutputSignals=longObjCalcInfo.LongObjRawInfo.ObjectTypeCameraConfirmed,longObjCalcInfo.longObjAbsInfo.dxFrontBumper,longObjCalcInfo.LongCollisionAssess.longCollisionSeverity,longObjCalcInfo.overlapRatio,LCK_Active,longObjCalcInfo.probEscape,subjInfo.subjCurvTraj
  vehAEBfullObjRelActiveCondCalc : Subsystem
  vehAEBfullSituationRelActiveCondCalc : Subsystem
  vehAEBfullSuppCondFinalJudge : Subsystem
  vehAEBfullVehRelActiveCondCalc : Subsystem
  vehAEBfullSuppCondFulfilled : Outport
  wires:
  Bus Selector1/6 -> vehAEBfullVehRelActiveCondCalc/6(VehConfigIbooster)  "<VehConfigIbooster>"
  Bus Selector/17 -> vehAEBfullObjRelActiveCondCalc/17(ObjFusQuality)  "<ObjFusQuality>"
  Bus Selector2/5 -> vehAEBfullSituationRelActiveCondCalc/5(LCK_Active)  "<LCK_Active>"
  Bus Selector2/7 -> vehAEBfullSituationRelActiveCondCalc/7(subjCurvTraj)  "<subjCurvTraj>"
  Bus Selector2/6 -> vehAEBfullSituationRelActiveCondCalc/6(probEscape)  "<probEscape>"
  Bus Selector2/4 -> vehAEBfullSituationRelActiveCondCalc/4(overlapRatio)  "<overlapRatio>"
  Bus Selector2/3 -> vehAEBfullSituationRelActiveCondCalc/3(longCollisionSeverity)  "<longCollisionSeverity>"
  Bus Selector2/2 -> vehAEBfullSituationRelActiveCondCalc/2(dxFrontBumper)  "<dxFrontBumper>"
  Bus Selector2/1 -> vehAEBfullSituationRelActiveCondCalc/1(ObjectTypeCameraConfirmed)  "<ObjectTypeCameraConfirmed>"
  Bus Selector1/11 -> vehAEBfullVehRelActiveCondCalc/11(AccrPedlPosnDiagc)  "<AccrPedlPosnDiagc>"
  Bus Selector1/10 -> vehAEBfullVehRelActiveCondCalc/10(subjVel)  "<subjVel>"
  Bus Selector1/9 -> vehAEBfullVehRelActiveCondCalc/9(subjLongAccel)  "<subjLongAccel>"
  Bus Selector1/8 -> vehAEBfullVehRelActiveCondCalc/8(BrakePedalStatus_G)  "<BrakePedalStatus_G>"
  Bus Selector1/7 -> vehAEBfullVehRelActiveCondCalc/7(ESPmasterCylindBrakePress)  "<ESP_MasterCylindBrakePress>"
  Bus Selector1/5 -> vehAEBfullVehRelActiveCondCalc/5(SteerWheelSpd)  "<SteerWheelSpd>"
  Bus Selector1/4 -> vehAEBfullVehRelActiveCondCalc/4(SteerWheelAngle)  "<SteerWheelAngle>"
  Bus Selector1/3 -> vehAEBfullVehRelActiveCondCalc/3(vehStateCalcAEBfullSuppFlg)  "<VSCvehAEBfullSuppCond>"
  Bus Selector1/2 -> vehAEBfullVehRelActiveCondCalc/2(ObjectMovingState)  "<ObjectMovingState>"
  Bus Selector1/1 -> vehAEBfullVehRelActiveCondCalc/1(ObjectTypeCameraConfirmed)  "<ObjectTypeCameraConfirmed>"
  Bus Selector/16 -> vehAEBfullObjRelActiveCondCalc/16(dxVariance)  "<dxVariance>"
  Bus Selector/15 -> vehAEBfullObjRelActiveCondCalc/15(ObjectIsObstacleProb)  "<ObjectIsObstacleProb>"
  Bus Selector/1 -> vehAEBfullObjRelActiveCondCalc/1(ObjectMovingState)  "<ObjectMovingState>"
  Bus Selector/14 -> vehAEBfullObjRelActiveCondCalc/14(ObjectExistProb)  "<ObjectExistProb>"
  Bus Selector/2 -> vehAEBfullObjRelActiveCondCalc/2(dz)  "<dz>"
  Bus Selector/13 -> vehAEBfullObjRelActiveCondCalc/13(ObjectID)  "<ObjectID>"
  Bus Selector/3 -> vehAEBfullObjRelActiveCondCalc/3(dxFrontBumper)  "<dxFrontBumper>"
  Bus Selector/12 -> vehAEBfullObjRelActiveCondCalc/12(ObjectMovingOrientation)  "<ObjectMovingOrientation>"
  Bus Selector/4 -> vehAEBfullObjRelActiveCondCalc/4(ObjectTypeCameraConfirmed)  "<ObjectTypeCameraConfirmed>"
  Bus Selector/11 -> vehAEBfullObjRelActiveCondCalc/11(AEB_PedResp)  "<AEB_PedResp>"
  Bus Selector/5 -> vehAEBfullObjRelActiveCondCalc/5(ObjectType)  "<ObjectType>"
  Bus Selector/10 -> vehAEBfullObjRelActiveCondCalc/10(vehConfigAEBPed)  "<VehicleConfigAEBPed>"
  Bus Selector/6 -> vehAEBfullObjRelActiveCondCalc/6(subjCurvTraj)  "<subjCurvTraj>"
  Bus Selector/9 -> vehAEBfullObjRelActiveCondCalc/9(vehConfigAEBCyc)  "<VehicleConfigAEBCyc>"
  Bus Selector/7 -> vehAEBfullObjRelActiveCondCalc/7(subjLatAccel)  "<subjLatAccel>"
  Bus Selector/8 -> vehAEBfullObjRelActiveCondCalc/8(subjVel)  "<subjVel>"
  inputSignals/1 -> Bus Selector/1, Bus Selector1/1, Bus Selector2/1
  vehAEBfullSuppCondFinalJudge/1(vehAEBfullSuppCondFulfilled) -> vehAEBfullSuppCondFulfilled/1  "vehAEBfullSuppCondFulfilled"
  vehAEBfullVehRelActiveCondCalc/1(vehAEBfullVehRelActiveCondFulfilled) -> vehAEBfullSuppCondFinalJudge/2(vehAEBfullVehRelActiveCondFulfilled)  "AEB_vehAEBfullVehRelActiveCond"
  vehAEBfullObjRelActiveCondCalc/1(vehAEBfullObjRelActiveCondFulfilled) -> vehAEBfullSuppCondFinalJudge/1(vehAEBfullObjRelActiveCondFulfilled)  "AEB_vehAEBfullObjRelActiveCond"
  vehAEBfullSituationRelActiveCondCalc/1(vehAEBfullSituationRelActiveCondFulfilled) -> vehAEBfullSuppCondFinalJudge/3(vehAEBfullSituationRelActiveCondFulfilled)  "AEB_vehAEBfullSitRelActiveCond"

== system TosAeb/FcwAebJa_Step_sys/ALG_AEBSystem/ALG_AEBController/AEB_FuncCondCalcApp/LongFunctionCondCalc/vehAEBfullSuppAborCondCalc/vehAEBfullAborCondCalc ==
  inputSignals : Inport
  Bus Selector : BusSelector  OutputSignals=longObjCalcInfo.LongObjRawInfo.ObjectID,longObjCalcInfo.LongObjRawInfo.ObjectExistProb,longObjCalcInfo.LongObjRawInfo.ObjectIsObstacleProb,longObjCalcInfo.LongObjRawInfo.dxVariance
  Bus Selector1 : BusSelector  OutputSignals=vehCanInfo.SteerWheelAngle,vehCanInfo.SteerWheelSpd,vehCanInfo.AccrPedlPosnDiagc,vehStateCalcInfo.VSCVehSuppAborCond.VSCvehAEBfullAborCond
  Bus Selector2 : BusSelector  OutputSignals=longObjCalcInfo.overlapRatio,longObjCalcInfo.longObjAbsInfo.dxFrontBumper,longObjCalcInfo.probEscape,longObjCalcInfo.LongObjRawInfo.ObjectMovingState
  vehAEBfullAborCondFinalJudge : Subsystem
  vehAEBfullObjRelAborCondCalc : Subsystem
  vehAEBfullSituationRelAborCondCalc : Subsystem
  vehAEBfullVehRelAborCondCalc : Subsystem
  vehAEBfullAborCondNoStandstillFulfilled : Outport
  vehAEBfullAborCondStandstillFulfilled : Outport  Port=2
  wires:
  Bus Selector2/4 -> vehAEBfullSituationRelAborCondCalc/4(ObjectMovingState)  "<ObjectMovingState>"
  Bus Selector2/3 -> vehAEBfullSituationRelAborCondCalc/3(probEscape)  "<probEscape>"
  Bus Selector2/2 -> vehAEBfullSituationRelAborCondCalc/2(dxFrontBumper)  "<dxFrontBumper>"
  Bus Selector2/1 -> vehAEBfullSituationRelAborCondCalc/1(overlapRatio)  "<overlapRatio>"
  Bus Selector1/4 -> vehAEBfullVehRelAborCondCalc/4(vehStateCalcAEBfullAborFlg)  "<VSCvehAEBfullAborCond>"
  Bus Selector1/3 -> vehAEBfullVehRelAborCondCalc/3(AccrPedlPosnDiagc)  "<AccrPedlPosnDiagc>"
  Bus Selector1/2 -> vehAEBfullVehRelAborCondCalc/2(SteerWheelSpd)  "<SteerWheelSpd>"
  Bus Selector1/1 -> vehAEBfullVehRelAborCondCalc/1(SteerWheelAngle)  "<SteerWheelAngle>"
  Bus Selector/4 -> vehAEBfullObjRelAborCondCalc/4(dxVariance)  "<dxVariance>"
  Bus Selector/3 -> vehAEBfullObjRelAborCondCalc/3(ObjectIsObstacleProb)  "<ObjectIsObstacleProb>"
  Bus Selector/2 -> vehAEBfullObjRelAborCondCalc/2(ObjectExistProb)  "<ObjectExistProb>"
  Bus Selector/1 -> vehAEBfullObjRelAborCondCalc/1(ObjectID)  "<ObjectID>"
  inputSignals/1 -> Bus Selector/1, Bus Selector1/1, Bus Selector2/1
  vehAEBfullObjRelAborCondCalc/1(vehAEBfullObjRelAborCondFulfilled) -> vehAEBfullAborCondFinalJudge/1(vehAEBfullObjRelAborCondFulfilled)  "AEB_vehAEBfullObjRelAborCond"
  vehAEBfullVehRelAborCondCalc/1(vehAEBfullVehRelAborCondFulfilled) -> vehAEBfullAborCondFinalJudge/2(vehAEBfullVehRelAborCondFulfilled)  "AEB_vehAEBfullVehRelAborCond"
  vehAEBfullSituationRelAborCondCalc/1(vehAEBfullSituationRelAborCondFulfilled) -> vehAEBfullAborCondFinalJudge/3(vehAEBfullSituationRelAborCondFulfilled)  "AEB_vehAEBfullSitRelAborCond"
  vehAEBfullAborCondFinalJudge/1(vehAEBfullAborCondNoStandstillFulfilled) -> vehAEBfullAborCondNoStandstillFulfilled/1
  vehAEBfullAborCondFinalJudge/2(vehAEBfullAborCondStandstillFulfilled) -> vehAEBfullAborCondStandstillFulfilled/1

== system TosAeb/FcwAebJa_Step_sys/ALG_AEBSystem/ALG_AEBController/AEB_FuncCondCalcApp/LongFunctionCondCalc/vehAEBpartSuppAborCondCalc/vehAEBpartAborCondCalc ==
  inputSignals : Inport
  Bus Selector : BusSelector  OutputSignals=longObjCalcInfo.LongObjRawInfo.ObjectID,longObjCalcInfo.LongObjRawInfo.ObjectExistProb,longObjCalcInfo.LongObjRawInfo.ObjectIsObstacleProb
  Bus Selector1 : BusSelector  OutputSignals=vehCanInfo.SteerWheelAngle,vehCanInfo.SteerWheelSpd,vehCanInfo.AccrPedlPosnDiagc,vehStateCalcInfo.VSCVehSuppAborCond.VSCvehAEBpartAborCond
  Bus Selector2 : BusSelector  OutputSignals=longObjCalcInfo.overlapRatio,longObjCalcInfo.longObjAbsInfo.dxFrontBumper,longObjCalcInfo.probEscape
  vehAEBpartAborCondFinalJudge : Subsystem
  vehAEBpartObjRelAborCondCalc : Subsystem
  vehAEBpartSituationRelAborCondCalc : Subsystem
  vehAEBpartVehRelAborCondCalc : Subsystem
  vehAEBpartAborCondNoStandstillFulfilled : Outport
  vehAEBpartAborCondStandstillFulfilled : Outport  Port=2
  wires:
  Bus Selector2/3 -> vehAEBpartSituationRelAborCondCalc/3(probEscape)  "<probEscape>"
  Bus Selector2/2 -> vehAEBpartSituationRelAborCondCalc/2(dxFrontBumper)  "<dxFrontBumper>"
  Bus Selector2/1 -> vehAEBpartSituationRelAborCondCalc/1(overlapRatio)  "<overlapRatio>"
  Bus Selector1/4 -> vehAEBpartVehRelAborCondCalc/4(vehStateCalcAEBpartAborFlg)  "<VSCvehAEBpartAborCond>"
  Bus Selector1/3 -> vehAEBpartVehRelAborCondCalc/3(AccrPedlPosnDiagc)  "<AccrPedlPosnDiagc>"
  Bus Selector1/2 -> vehAEBpartVehRelAborCondCalc/2(SteerWheelSpd)  "<SteerWheelSpd>"
  Bus Selector1/1 -> vehAEBpartVehRelAborCondCalc/1(SteerWheelAngle)  "<SteerWheelAngle>"
  Bus Selector/3 -> vehAEBpartObjRelAborCondCalc/3(ObjectIsObstacleProb)  "<ObjectIsObstacleProb>"
  Bus Selector/1 -> vehAEBpartObjRelAborCondCalc/1(ObjectID)  "<ObjectID>"
  Bus Selector/2 -> vehAEBpartObjRelAborCondCalc/2(ObjectExistProb)  "<ObjectExistProb>"
  inputSignals/1 -> Bus Selector/1, Bus Selector1/1, Bus Selector2/1
  vehAEBpartAborCondFinalJudge/2(vehAEBpartAborCondStandstillFulfilled) -> vehAEBpartAborCondStandstillFulfilled/1
  vehAEBpartAborCondFinalJudge/1(vehAEBpartAborCondNoStandstillFulfilled) -> vehAEBpartAborCondNoStandstillFulfilled/1
  vehAEBpartSituationRelAborCondCalc/1(vehAEBpartSituationRelAborCondFulfilled) -> vehAEBpartAborCondFinalJudge/3(vehAEBpartSituationRelAborCondFulfilled)  "AEB_vehAEBpartSituationRelAborCond"
  vehAEBpartVehRelAborCondCalc/1(vehAEBpartVehRelAborCondFulfilled) -> vehAEBpartAborCondFinalJudge/2(vehAEBpartVehRelAborCondFulfilled)  "AEB_vehAEBpartVehRelAborCond"
  vehAEBpartObjRelAborCondCalc/1(vehAEBpartObjRelAborCondFulfilled) -> vehAEBpartAborCondFinalJudge/1(vehAEBpartObjRelAborCondFulfilled)  "AEB_vehAEBpartObjRelAborCond"
